simulink library "ee_lib_for_power_systems"  (R2025b) (continued)
== system ee_lib_for_power_systems/Power Converters/Thyristor Bridge (Three-Phase) ==
  g : Inport
  Demux : Demux  Outputs=6
  Simulink-PS Converter{,1..5} (6 alike) : nesl_utility/Simulink-PS Converter  PhysicalDomain=network_engine_domain  SubClassName=ps_input  LeftPortType=input  RightPortType=output  PseudoPeriodic=off  Frequencies=[]  NoiseDistribution=none  NoiseParameters=[]  Unit=1  AffineConversion=off  FilteringAndDerivatives=zero  SimscapeFilterOrder=1  InputFilterTimeConstant=0.001  UdotUserProvided=0
  Six-Pulse Gate Multiplexer : ee_lib/Semiconductors & Converters/Converters/Six-Pulse Gate Multiplexer  port_option=ee.enum.controlport.ps
  converter : ee_lib/Semiconductors & Converters/Converters/Converter (Three-Phase)  port_option=ee.enum.threePhasePort.composite  fidelity_option=ee.enum.converters.fidelity.detailed  device_type=ee.enum.converters.switchingdevice.thyristor  showThermalPort=false  K=0  Vth=0.5 V  Vgt=0.5 V  Vgt_off=0.4 V  Ih=1e-3 A  Vf=Vfs V  Ron=Ron Ohm  Rds=Ron Ohm  Goff=Goff 1/Ohm  Eon_scalar=22.86e-3 J  Eoff_scalar=17.14e-3 J  Eoff_natural_commutation=10e-3 J  Voff=300 V  Iout_scalar=600 A  onCurrentMeasDelay=0 s  useCurrentFromPreviousCycle=false  useVoltageFromPreviousCycle=false  diode_param=ee.enum.converters.protectiondiode.none  diode_Vf=Vfd V  diode_Ron=Ron Ohm  diode_Goff=Goff 1/Ohm  diode_C=50 nF  diode_iRM=-235 A  diode_iF=300 A  diode_idot=-50 A/us  diode_trr_param=ee.enum.converters.diodetrr.trr_factor  diode_trr_factor=3  diode_trr=15 us  diode_Qrr=1500 s*uA  diode_rec_loss_model=ee.enum.diode.reverseRecoveryModel.fixed  diode_ErecVal=0 J  diode_scaleWithIandV=false  diode_ErecMat=zeros(2, 3) J  diode_TjrecVec=[298.15, 398.15] K  diode_IfrecVec=[.1, 1, 10] A  diode_IrecVal=10 A  diode_VrecVal=10 V  diode_tFilter=1 / 1000 / 100 s  thermal_network_parameterization=ee.enum.thermalNetworkTopology.external  Rth=[.08, .5] K/W  Rth_Cauer=[.08, .1, .5] K/W  Rth_Foster=[.08, .14, .22, .16] K/W  thermal_mass_parameterization=ee.enum.thermalNetwork.timeconstant  thermal_mass=[.01, .5] J/K  thermal_mass_Cauer=[.01, .1, .5] J/K  thermal_mass_Foster=[.001, .005, .05, .5] J/K  thermal_time_constant=[.001, .2] s  thermal_time_constant_Cauer=[.001, .1, .2] s  thermal_time_constant_Foster=[7e-05, .0007, .01, .08] s  thermal_mass_initial_temperatures=[25, 25] degC  thermal_mass_initial_temperatures_Cauer=[25, 25, 25] degC  junction_thermal_mass=0.01 J/K  Tvec_initial_Foster=[25, 25, 25, 25] degC  snubber_type=ee.enum.converters.snubber.exclude  Cs=1e-7 F  Rs=0.1 Ohm  Vce_loss=[0, 150, 300] V
  + : PMIOPort  Side=Right
  "~ " : PMIOPort  Port=2  Side=Left
  - : PMIOPort  Port=3  Side=Right
  wires:
  Demux/6 -> Simulink-PS Converter2/1
  Demux/5 -> Simulink-PS Converter5/1
  Demux/4 -> Simulink-PS Converter/1
  Demux/3 -> Simulink-PS Converter3/1
  Demux/2 -> Simulink-PS Converter4/1
  Demux/1 -> Simulink-PS Converter1/1
  g/1 -> Demux/1
  connections:
  Simulink-PS Converter2/RConn1 -- Six-Pulse Gate Multiplexer/LConn3
  Simulink-PS Converter5/RConn1 -- Six-Pulse Gate Multiplexer/LConn6
  Simulink-PS Converter1/RConn1 -- Six-Pulse Gate Multiplexer/LConn2
  converter/LConn2 -- "~ "/RConn1
  Simulink-PS Converter3/RConn1 -- Six-Pulse Gate Multiplexer/LConn4
  Simulink-PS Converter/RConn1 -- Six-Pulse Gate Multiplexer/LConn1
  Simulink-PS Converter4/RConn1 -- Six-Pulse Gate Multiplexer/LConn5
  converter/LConn1 -- Six-Pulse Gate Multiplexer/RConn1
  -/RConn1 -- converter/RConn1
  +/RConn1 -- converter/RConn2

== system ee_lib_for_power_systems/Power Converters/Two-Level Average-Switch ==
  g : Inport
  Average-Value Voltage Source Converter (Three-Phase) : ee_lib/Semiconductors & Converters/Converters/Average-Value Voltage Source Converter (Three-Phase)  port_option=ee.enum.threePhasePort.composite  frequency_option=ee.enum.converters.frequency.variable  input_option=ee.enum.converters.inputOption.ModWave  FRated=60 Hz  losses_parameterization=ee.enum.converters.losses.fixed  Pfixed=1 W  ks=0.01  kc1=1.7 V  kc2=0.01 Ohm  Ploss_vect=[100, 800, 1870, 3250, 4900] W  Irms_vect=[10, 100, 200, 300, 400] A  Vdc_nom=300 V  thermal_mass=30000 J/K  temperature_specify=off  temperature_priority=High  temperature=298.15 K  temperature_nominal_specify=off  temperature_nominal_value=1  temperature_nominal_unit=K
      settings: InternalSimscapePortConfiguration="{\"Bottom\":[],\"Left\":[{\"id\":\"ModWave\",\"label\":\"ModWave\",\"type\":\"input\"},{\"id\":\"N\",\"label\":\"~\",\"type\":\"foundation.electrical.three_phase\"}],\"Right\":[{\"id\":\"p\",\"label\":\"+\",\"type\":\"foundation.electrical.electrical\"},{\"id\":\"n\",\"label\":\"-\",\"type\":\"foundation.electrical.electrical\"}],\"Top\":[]}"
  Demux : Demux  Outputs=6
  Mux : Mux  Inputs=3
  Simulink-PS Converter : nesl_utility/Simulink-PS Converter  PhysicalDomain=network_engine_domain  SubClassName=ps_input  LeftPortType=input  RightPortType=output  PseudoPeriodic=off  Frequencies=[]  NoiseDistribution=none  NoiseParameters=[]  Unit=1  AffineConversion=off  FilteringAndDerivatives=provide  SimscapeFilterOrder=1  InputFilterTimeConstant=0.001  UdotUserProvided=0
  Sum : Sum  Inputs=|+-
  Sum1 : Sum  Inputs=|+-
  Sum2 : Sum  Inputs=|+-
  + : PMIOPort  Side=Left
  - : PMIOPort  Port=2  Side=Left
  ~ : PMIOPort  Port=3  Side=Right
  wires:
  Mux/1 -> Simulink-PS Converter/1
  Sum2/1 -> Mux/3
  Sum1/1 -> Mux/2
  Sum/1 -> Mux/1
  Demux/6 -> Sum2/2
  Demux/5 -> Sum2/1
  Demux/4 -> Sum1/2
  Demux/3 -> Sum1/1
  Demux/2 -> Sum/2
  Demux/1 -> Sum/1
  g/1 -> Demux/1
  connections:
  Simulink-PS Converter/RConn1 -- Average-Value Voltage Source Converter (Three-Phase)/LConn1
  ~/RConn1 -- Average-Value Voltage Source Converter (Three-Phase)/LConn2
  +/RConn1 -- Average-Value Voltage Source Converter (Three-Phase)/RConn1
  -/RConn1 -- Average-Value Voltage Source Converter (Three-Phase)/RConn2

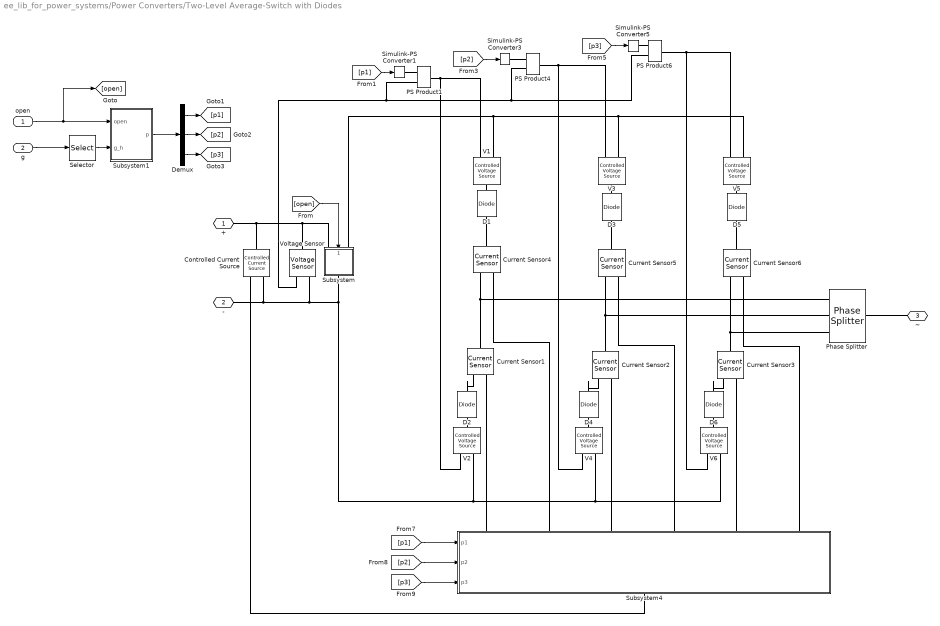
== system ee_lib_for_power_systems/Power Converters/Two-Level Average-Switch with Diodes ==
  open : Inport
  g : Inport  Port=2
  Controlled Current Source : fl_lib/Electrical/Electrical Sources/Controlled Current Source
  Current Sensor{1..6} (6 alike) : fl_lib/Electrical/Electrical Sensors/Current Sensor
  D{1..6} (6 alike) : fl_lib/Electrical/Electrical Elements/Diode  Vf=Vf V  Ron=Ron Ohm  Goff=Goff 1/Ohm  i_specify=off  i_priority=None  i=0 A  i_nominal_specify=off  i_nominal_value=1  i_nominal_unit=A  v_specify=off  v_priority=None  v=0 V  v_nominal_specify=off  v_nominal_value=1  v_nominal_unit=V
  Demux : Demux  Outputs=3
  PS Product1 : fl_lib/Physical Signals/Functions/PS Product
  PS Product4 : fl_lib/Physical Signals/Functions/PS Product
  PS Product6 : fl_lib/Physical Signals/Functions/PS Product
  Phase Splitter : ee_lib/Connectors & References/Phase Splitter
  Selector : Selector  InputPortWidth=6  IndexOptions=Index vector (dialog)  Indices=[1 3 5]  OutputSizes=1
  Simulink-PS Converter1 : nesl_utility/Simulink-PS Converter  PhysicalDomain=network_engine_domain  SubClassName=ps_input  LeftPortType=input  RightPortType=output  PseudoPeriodic=off  Frequencies=[]  NoiseDistribution=none  NoiseParameters=[]  Unit=1  AffineConversion=off  FilteringAndDerivatives=provide  SimscapeFilterOrder=1  InputFilterTimeConstant=0.001  UdotUserProvided=0
  Simulink-PS Converter3 : nesl_utility/Simulink-PS Converter  PhysicalDomain=network_engine_domain  SubClassName=ps_input  LeftPortType=input  RightPortType=output  PseudoPeriodic=off  Frequencies=[]  NoiseDistribution=none  NoiseParameters=[]  Unit=1  AffineConversion=off  FilteringAndDerivatives=provide  SimscapeFilterOrder=1  InputFilterTimeConstant=0.001  UdotUserProvided=0
  Simulink-PS Converter5 : nesl_utility/Simulink-PS Converter  PhysicalDomain=network_engine_domain  SubClassName=ps_input  LeftPortType=input  RightPortType=output  PseudoPeriodic=off  Frequencies=[]  NoiseDistribution=none  NoiseParameters=[]  Unit=1  AffineConversion=off  FilteringAndDerivatives=provide  SimscapeFilterOrder=1  InputFilterTimeConstant=0.001  UdotUserProvided=0
  Subsystem : Subsystem
      settings: PortSchema="{\"entries\":[{\"content\":{\"sides\":[{\"content\":{},\"type\":\"ConnectorPlacement.EquallySpacedRectSide\",\"uuid\":\"88db1bd8-f64b-4165-8d2c-7cfa4014cba2\"},{\"content\":{\"side\":\"RIGHT\"},\"type\":\"ConnectorPlacement.EquallySpacedRectSide\",\"uuid\":\"19f0c499-9eed-48d3-a8f5-fe9dad581b85\"},{\"content\":{\"connectorIds\":[\"LConn1\",\"In1\",\"LConn2\"],\"side\":\"TOP\"},\"type\":\"ConnectorPlacement.EquallySpacedRectSide\",\"uuid\":\"71d5fe89-ffc2-4430-819d-512bfd197a1c\"},{\"content\":{\"connectorIds\":[\"RConn1\"],\"side\":\"BOTTOM\"},\"type\":\"ConnectorPlacement.EquallySpacedRectSide\",\"uuid\":\"b828cb58-5f78-4b23-b8a0-147c7a9d81e7\"}]},\"type\":\"ConnectorPlacement.EquallySpacedConnector\",\"uuid\":\"64b174d4-80a0-4eb7-9a75-e3677787263f\"}],\"packageUris\":[\"http://schema.mathworks.com/mf0/Connector/R2020b\"],\"version\":\"1.0\"}"
  Subsystem1 : Subsystem
  Subsystem4 : Subsystem
      settings: PortSchema="{\"entries\":[{\"content\":{\"sides\":[{\"content\":{\"connectorIds\":[\"LConn1\",\"LConn2\",\"LConn3\",\"LConn4\",\"LConn5\",\"LConn6\"]},\"type\":\"ConnectorPlacement.EquallySpacedRectSide\",\"uuid\":\"5fd9aafb-b495-44e2-9499-8cdfe2ab4f56\"},{\"content\":{\"connectorIds\":[\"RConn1\"],\"side\":\"RIGHT\"},\"type\":\"ConnectorPlacement.EquallySpacedRectSide\",\"uuid\":\"0d8ceae3-09aa-4016-97de-ee2249771a91\"},{\"content\":{\"connectorIds\":[\"In1\",\"In2\",\"In3\"],\"side\":\"TOP\"},\"type\":\"ConnectorPlacement.EquallySpacedRectSide\",\"uuid\":\"0b4c8ea8-b524-451d-8365-47bbe880e547\"}]},\"type\":\"ConnectorPlacement.EquallySpacedConnector\",\"uuid\":\"44c8dc65-a8bd-4e02-863f-9a87b0ac0f96\"}],\"packageUris\":[\"http://schema.mathworks.com/mf0/Connector/R2020b\"],\"version\":\"1.0\"}"
  V{1..6} (6 alike) : fl_lib/Electrical/Electrical Sources/Controlled Voltage Source
  Voltage Sensor : fl_lib/Electrical/Electrical Sensors/Voltage Sensor
  + : PMIOPort  Side=Left
  - : PMIOPort  Port=2  Side=Left
  ~ : PMIOPort  Port=3  Side=Right
  wires:
  open/1 -> [open], Subsystem1/1(open)
  g/1 -> Selector/1
  Selector/1 -> Subsystem1/2(g_h)
  Subsystem1/1(p) -> Demux/1
  [open] -> Subsystem/1(open)
  Demux/1 -> [p1]
  Demux/2 -> [p2]
  Demux/3 -> [p3]
  [p1] -> Simulink-PS Converter1/1
  [p2] -> Simulink-PS Converter3/1
  [p3] -> Simulink-PS Converter5/1
  [p1] -> Subsystem4/1(p1)
  [p2] -> Subsystem4/2(p2)
  [p3] -> Subsystem4/3(p3)
  connections:
  Subsystem/LConn1 -- Controlled Current Source/LConn1 -- +/RConn1 -- Voltage Sensor/LConn1
  D2/LConn1 -- V2/LConn1
  PS Product1/RConn1 -- V2/RConn1 -- V1/RConn1
  Simulink-PS Converter1/RConn1 -- PS Product1/LConn1
  D2/RConn1 -- Current Sensor1/RConn2
  PS Product1/LConn2 -- Voltage Sensor/RConn1 -- PS Product6/LConn2 -- PS Product4/LConn2
  Current Sensor1/LConn1 -- Current Sensor4/RConn2 -- Phase Splitter/RConn1
  V1/LConn1 -- D1/RConn1
  Subsystem/RConn1 -- V6/RConn2 -- V4/RConn2 -- V2/RConn2 -- Controlled Current Source/RConn2 -- -/RConn1 -- Voltage Sensor/RConn2
  Subsystem/LConn2 -- V5/RConn2 -- V3/RConn2 -- V1/RConn2
  Phase Splitter/RConn2 -- Current Sensor5/RConn2 -- Current Sensor2/LConn1
  D3/RConn1 -- V3/LConn1
  D4/RConn1 -- Current Sensor2/RConn2
  Simulink-PS Converter3/RConn1 -- PS Product4/LConn1
  D4/LConn1 -- V4/LConn1
  PS Product4/RConn1 -- V3/RConn1 -- V4/RConn1
  Phase Splitter/RConn3 -- Current Sensor6/RConn2 -- Current Sensor3/LConn1
  D6/LConn1 -- V6/LConn1
  D6/RConn1 -- Current Sensor3/RConn2
  PS Product6/RConn1 -- V6/RConn1 -- V5/RConn1
  Simulink-PS Converter5/RConn1 -- PS Product6/LConn1
  D5/RConn1 -- V5/LConn1
  ~/RConn1 -- Phase Splitter/LConn1
  Current Sensor4/LConn1 -- D1/LConn1
  D3/LConn1 -- Current Sensor5/LConn1
  D5/LConn1 -- Current Sensor6/LConn1
  Subsystem4/LConn2 -- Current Sensor4/RConn1
  Subsystem4/LConn4 -- Current Sensor5/RConn1
  Subsystem4/LConn6 -- Current Sensor6/RConn1
  Subsystem4/RConn1 -- Controlled Current Source/RConn1
  Subsystem4/LConn1 -- Current Sensor1/RConn1
  Subsystem4/LConn3 -- Current Sensor2/RConn1
  Subsystem4/LConn5 -- Current Sensor3/RConn1

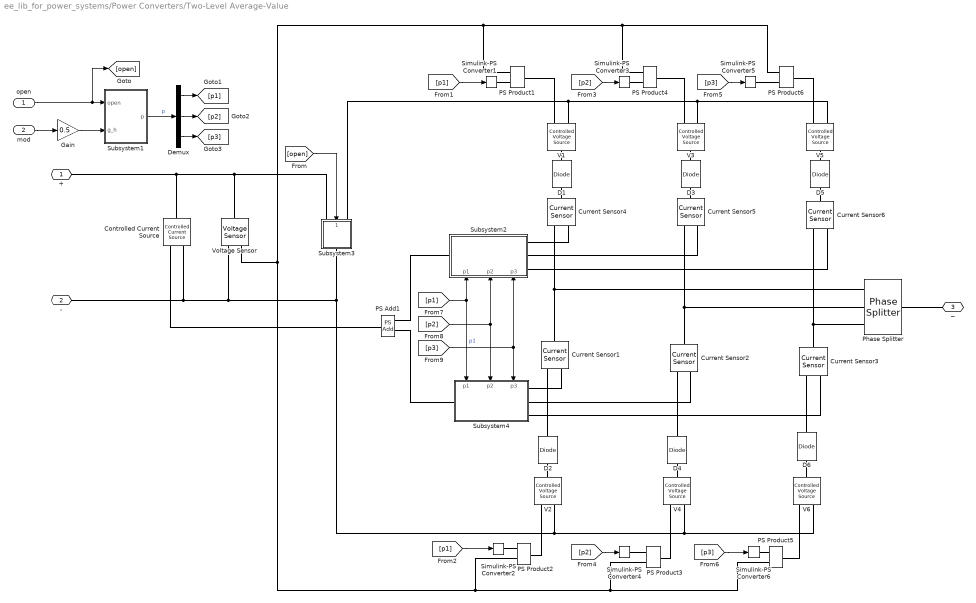
== system ee_lib_for_power_systems/Power Converters/Two-Level Average-Value ==
  open : Inport
  mod : Inport  Port=2
  Controlled Current Source : fl_lib/Electrical/Electrical Sources/Controlled Current Source
  Current Sensor{1..6} (6 alike) : fl_lib/Electrical/Electrical Sensors/Current Sensor
  D{1..6} (6 alike) : fl_lib/Electrical/Electrical Elements/Diode  Vf=Vf V  Ron=Ron Ohm  Goff=Goff 1/Ohm  i_specify=off  i_priority=None  i=0 A  i_nominal_specify=off  i_nominal_value=1  i_nominal_unit=A  v_specify=off  v_priority=None  v=0 V  v_nominal_specify=off  v_nominal_value=1  v_nominal_unit=V
  Demux : Demux  Outputs=3
  Gain : Gain  Gain=0.5
  PS Add1 : fl_lib/Physical Signals/Functions/PS Add
  PS Product{1..6} (6 alike) : fl_lib/Physical Signals/Functions/PS Product
  Phase Splitter : ee_lib/Connectors & References/Phase Splitter
  Simulink-PS Converter{1..6} (6 alike) : nesl_utility/Simulink-PS Converter  PhysicalDomain=network_engine_domain  SubClassName=ps_input  LeftPortType=input  RightPortType=output  PseudoPeriodic=off  Frequencies=[]  NoiseDistribution=none  NoiseParameters=[]  Unit=1  AffineConversion=off  FilteringAndDerivatives=provide  SimscapeFilterOrder=1  InputFilterTimeConstant=0.001  UdotUserProvided=0
  Subsystem1 : Subsystem
  Subsystem2 : Subsystem
      settings: PortSchema="{\"entries\":[{\"content\":{\"sides\":[{\"content\":{\"connectorIds\":[\"LConn1\",\"LConn2\",\"LConn3\"]},\"type\":\"ConnectorPlacement.EquallySpacedRectSide\",\"uuid\":\"5fd9aafb-b495-44e2-9499-8cdfe2ab4f56\"},{\"content\":{\"connectorIds\":[\"RConn1\"],\"side\":\"RIGHT\"},\"type\":\"ConnectorPlacement.EquallySpacedRectSide\",\"uuid\":\"0d8ceae3-09aa-4016-97de-ee2249771a91\"},{\"content\":{\"side\":\"TOP\"},\"type\":\"ConnectorPlacement.EquallySpacedRectSide\",\"uuid\":\"0b4c8ea8-b524-451d-8365-47bbe880e547\"},{\"content\":{\"connectorIds\":[\"In1\",\"In2\",\"In3\"],\"side\":\"BOTTOM\"},\"type\":\"ConnectorPlacement.EquallySpacedRectSide\",\"uuid\":\"91c10802-5411-4afb-b6a0-ea8a924d9d4e\"}]},\"type\":\"ConnectorPlacement.EquallySpacedConnector\",\"uuid\":\"44c8dc65-a8bd-4e02-863f-9a87b0ac0f96\"}],\"packageUris\":[\"http://schema.mathworks.com/mf0/Connector/R2020b\"],\"version\":\"1.0\"}"
  Subsystem3 : Subsystem
      settings: PortSchema="{\"entries\":[{\"content\":{\"sides\":[{\"content\":{},\"type\":\"ConnectorPlacement.EquallySpacedRectSide\",\"uuid\":\"88db1bd8-f64b-4165-8d2c-7cfa4014cba2\"},{\"content\":{\"side\":\"RIGHT\"},\"type\":\"ConnectorPlacement.EquallySpacedRectSide\",\"uuid\":\"19f0c499-9eed-48d3-a8f5-fe9dad581b85\"},{\"content\":{\"connectorIds\":[\"LConn1\",\"In1\",\"LConn2\"],\"side\":\"TOP\"},\"type\":\"ConnectorPlacement.EquallySpacedRectSide\",\"uuid\":\"71d5fe89-ffc2-4430-819d-512bfd197a1c\"},{\"content\":{\"connectorIds\":[\"RConn1\"],\"side\":\"BOTTOM\"},\"type\":\"ConnectorPlacement.EquallySpacedRectSide\",\"uuid\":\"b828cb58-5f78-4b23-b8a0-147c7a9d81e7\"}]},\"type\":\"ConnectorPlacement.EquallySpacedConnector\",\"uuid\":\"64b174d4-80a0-4eb7-9a75-e3677787263f\"}],\"packageUris\":[\"http://schema.mathworks.com/mf0/Connector/R2020b\"],\"version\":\"1.0\"}"
  Subsystem4 : Subsystem
      settings: PortSchema="{\"entries\":[{\"content\":{\"sides\":[{\"content\":{\"connectorIds\":[\"LConn1\",\"LConn2\",\"LConn3\"]},\"type\":\"ConnectorPlacement.EquallySpacedRectSide\",\"uuid\":\"5fd9aafb-b495-44e2-9499-8cdfe2ab4f56\"},{\"content\":{\"connectorIds\":[\"RConn1\"],\"side\":\"RIGHT\"},\"type\":\"ConnectorPlacement.EquallySpacedRectSide\",\"uuid\":\"0d8ceae3-09aa-4016-97de-ee2249771a91\"},{\"content\":{\"connectorIds\":[\"In1\",\"In2\",\"In3\"],\"side\":\"TOP\"},\"type\":\"ConnectorPlacement.EquallySpacedRectSide\",\"uuid\":\"0b4c8ea8-b524-451d-8365-47bbe880e547\"}]},\"type\":\"ConnectorPlacement.EquallySpacedConnector\",\"uuid\":\"44c8dc65-a8bd-4e02-863f-9a87b0ac0f96\"}],\"packageUris\":[\"http://schema.mathworks.com/mf0/Connector/R2020b\"],\"version\":\"1.0\"}"
  V{1..6} (6 alike) : fl_lib/Electrical/Electrical Sources/Controlled Voltage Source
  Voltage Sensor : fl_lib/Electrical/Electrical Sensors/Voltage Sensor
  + : PMIOPort  Side=Left
  - : PMIOPort  Port=2  Side=Left
  ~ : PMIOPort  Port=3  Side=Right
  wires:
  [p3] -> Simulink-PS Converter6/1
  [p2] -> Simulink-PS Converter4/1
  [p1] -> Simulink-PS Converter2/1
  [p3] -> Simulink-PS Converter5/1
  [p2] -> Simulink-PS Converter3/1
  [p1] -> Simulink-PS Converter1/1
  Demux/3 -> [p3]
  Demux/2 -> [p2]
  Demux/1 -> [p1]
  [open] -> Subsystem3/1(open)
  Subsystem1/1(p) -> Demux/1  "p"
  mod/1 -> Gain/1
  open/1 -> Subsystem1/1(open), [open]
  [p1] -> Subsystem2/3(p1), Subsystem4/3(p1)  "p1"
  [p2] -> Subsystem2/2(p2), Subsystem4/2(p2)
  [p3] -> Subsystem2/1(p3), Subsystem4/1(p3)
  Gain/1 -> Subsystem1/2(g_h)
  connections:
  Subsystem2/RConn1 -- PS Add1/LConn2
  Subsystem4/RConn1 -- PS Add1/LConn1
  Voltage Sensor/RConn2 -- -/RConn1 -- Controlled Current Source/RConn2 -- Subsystem3/RConn1 -- V4/RConn2 -- V6/RConn2 -- V2/RConn2
  PS Product6/LConn2 -- Simulink-PS Converter5/RConn1
  Simulink-PS Converter3/RConn1 -- PS Product4/LConn2
  Simulink-PS Converter1/RConn1 -- PS Product1/LConn2
  PS Product5/RConn1 -- V6/RConn1
  PS Product3/RConn1 -- V4/RConn1
  PS Product2/RConn1 -- V2/RConn1
  Simulink-PS Converter6/RConn1 -- PS Product5/LConn1
  Simulink-PS Converter2/RConn1 -- PS Product2/LConn1
  Simulink-PS Converter4/RConn1 -- PS Product3/LConn1
  Subsystem4/LConn3 -- Current Sensor3/RConn1
  Subsystem4/LConn2 -- Current Sensor2/RConn1
  Subsystem4/LConn1 -- Current Sensor1/RConn1
  D5/LConn1 -- Current Sensor6/LConn1
  D3/LConn1 -- Current Sensor5/LConn1
  Current Sensor4/LConn1 -- D1/LConn1
  D5/RConn1 -- V5/LConn1
  PS Product6/RConn1 -- V5/RConn1
  D6/RConn1 -- Current Sensor3/RConn2
  D6/LConn1 -- V6/LConn1
  Current Sensor3/LConn1 -- Current Sensor6/RConn2 -- Phase Splitter/RConn3
  PS Product4/RConn1 -- V3/RConn1
  D4/LConn1 -- V4/LConn1
  D4/RConn1 -- Current Sensor2/RConn2
  D3/RConn1 -- V3/LConn1
  Current Sensor2/LConn1 -- Current Sensor5/RConn2 -- Phase Splitter/RConn2
  V1/LConn1 -- D1/RConn1
  Current Sensor4/RConn2 -- Current Sensor1/LConn1 -- Phase Splitter/RConn1
  D2/RConn1 -- Current Sensor1/RConn2
  PS Product1/RConn1 -- V1/RConn1
  D2/LConn1 -- V2/LConn1
  Voltage Sensor/LConn1 -- +/RConn1 -- Controlled Current Source/LConn1 -- Subsystem3/LConn1
  Subsystem2/LConn1 -- Current Sensor4/RConn1
  Current Sensor5/RConn1 -- Subsystem2/LConn2
  Subsystem2/LConn3 -- Current Sensor6/RConn1
  PS Add1/RConn1 -- Controlled Current Source/RConn1
  V3/RConn2 -- V5/RConn2 -- Subsystem3/LConn2 -- V1/RConn2
  ~/RConn1 -- Phase Splitter/LConn1
  PS Product5/LConn2 -- PS Product3/LConn2 -- PS Product1/LConn1 -- PS Product4/LConn1 -- PS Product6/LConn1 -- Voltage Sensor/RConn1 -- PS Product2/LConn2

== system ee_lib_for_power_systems/Sense and Measure/Power sensor (Three-Phase) ==
  PS-Simulink Converter : nesl_utility/PS-Simulink Converter  PhysicalDomain=network_engine_domain  SubClassName=ps_output  LeftPortType=input  RightPortType=output  PseudoPeriodic=off  Frequencies=[]  VectorFormat=inherit  Unit=inherit  AffineConversion=off
  PS-Simulink Converter1 : nesl_utility/PS-Simulink Converter  PhysicalDomain=network_engine_domain  SubClassName=ps_output  LeftPortType=input  RightPortType=output  PseudoPeriodic=off  Frequencies=[]  VectorFormat=inherit  Unit=inherit  AffineConversion=off
  Power Sensor (Three-Phase) : ee_lib/Sensors & Transducers/Power Sensor (Three-Phase)  load_type=ee.enum.passive.type_balanced.balanced  f0=60 Hz
      settings: InternalSimscapePortConfiguration="{\"Bottom\":[],\"Left\":[{\"id\":\"N1\",\"label\":\"~1\",\"type\":\"foundation.electrical.three_phase\"}],\"Right\":[{\"id\":\"N2\",\"label\":\"~2\",\"type\":\"foundation.electrical.three_phase\"}],\"Top\":[{\"id\":\"Pm\",\"label\":\"P\",\"type\":\"output\"},{\"id\":\"Qm\",\"label\":\"Q\",\"type\":\"output\"}]}"
  ~1 : PMIOPort  Side=Left
  ~2 : PMIOPort  Port=2  Side=Right
  P : Outport
  Q : Outport  Port=2
  wires:
  PS-Simulink Converter1/1 -> Q/1
  PS-Simulink Converter/1 -> P/1
  connections:
  PS-Simulink Converter/LConn1 -- Power Sensor (Three-Phase)/LConn2
  Power Sensor (Three-Phase)/LConn1 -- ~1/RConn1
  PS-Simulink Converter1/LConn1 -- Power Sensor (Three-Phase)/LConn3
  Power Sensor (Three-Phase)/RConn1 -- ~2/RConn1

== system ee_lib_for_power_systems/Sense and Measure/VI sensor (Three-Phase) ==
  PS-Simulink Converter : nesl_utility/PS-Simulink Converter  PhysicalDomain=network_engine_domain  SubClassName=ps_output  LeftPortType=input  RightPortType=output  PseudoPeriodic=off  Frequencies=[]  VectorFormat=inherit  Unit=inherit  AffineConversion=off
  PS-Simulink Converter1 : nesl_utility/PS-Simulink Converter  PhysicalDomain=network_engine_domain  SubClassName=ps_output  LeftPortType=input  RightPortType=output  PseudoPeriodic=off  Frequencies=[]  VectorFormat=inherit  Unit=inherit  AffineConversion=off
  current_voltage : ee_lib/Sensors & Transducers/Current and Voltage Sensor (Three-Phase)  vMeasurementType=ee.enum.vmeasurement.lg  outputUnit=ee.enum.unit.si  SRated=Pnom A*V  VRated=Vnom V  I_specify=off  I_priority=None  I=[0, 0, 0] A  I_nominal_specify=off  I_nominal_value=1  I_nominal_unit=A  V_specify=off  V_priority=None  V=[0, 0, 0] V  V_nominal_specify=off  V_nominal_value=1  V_nominal_unit=V
      settings: InternalSimscapePortConfiguration="{\"Bottom\":[],\"Left\":[{\"id\":\"N1\",\"label\":\"~1\",\"type\":\"foundation.electrical.three_phase\"}],\"Right\":[{\"id\":\"V_output\",\"label\":\"V\",\"type\":\"output\"},{\"id\":\"I_output\",\"label\":\"I\",\"type\":\"output\"},{\"id\":\"N2\",\"label\":\"~2\",\"type\":\"foundation.electrical.three_phase\"}],\"Top\":[]}"
  ~1 : PMIOPort  Side=Left
  ~2 : PMIOPort  Port=2  Side=Right
  Vabc : Outport
  Iabc : Outport  Port=2
  wires:
  PS-Simulink Converter1/1 -> Iabc/1
  PS-Simulink Converter/1 -> Vabc/1
  connections:
  current_voltage/RConn3 -- ~2/RConn1
  PS-Simulink Converter/LConn1 -- current_voltage/RConn1
  current_voltage/LConn1 -- ~1/RConn1
  PS-Simulink Converter1/LConn1 -- current_voltage/RConn2

== system ee_lib_for_power_systems/Sense and Measure/Vectorized PQ meas ==
  Iabc : Inport
  Vabc : Inport  Port=2
  Product1 : Product  InputSameDT=on  SaturateOnIntegerOverflow=on  OutDataTypeStr=Inherit: Same as first input
  Product3 : Product  InputSameDT=on  SaturateOnIntegerOverflow=on  OutDataTypeStr=Inherit: Same as first input
  Sum1 : Sum  Inputs=1  CollapseMode=Specified dimension  InputSameDT=on  SaturateOnIntegerOverflow=on  OutDataTypeStr=Inherit: Same as first input
  Sum2 : Sum  Inputs=1  CollapseMode=Specified dimension  InputSameDT=on  SaturateOnIntegerOverflow=on  OutDataTypeStr=Inherit: Same as first input
  Sum4 : Sum  Inputs=+-  InputSameDT=on  SaturateOnIntegerOverflow=on  OutDataTypeStr=Inherit: Same as first input
  pu->V : Gain  Gain=1/sqrt(3)  SaturateOnIntegerOverflow=on  OutDataTypeStr=Inherit: Same as input  ParamDataTypeStr=Inherit: Same as input
  pu->V1 : Gain  Gain=[0 1 0;0 0 1;1 0 0]  Multiplication=Matrix(K*u)  SaturateOnIntegerOverflow=on  OutDataTypeStr=Inherit: Same as input  ParamDataTypeStr=Inherit: Same as input
  pu->V2 : Gain  Gain=[0 1 0;0 0 1;1 0 0]  Multiplication=Matrix(K*u)  SaturateOnIntegerOverflow=on  OutDataTypeStr=Inherit: Same as input  ParamDataTypeStr=Inherit: Same as input
  P : Outport  VectorParamsAs1DForOutWhenUnconnected=off
  Q : Outport  Port=2  VectorParamsAs1DForOutWhenUnconnected=off
  wires:
  pu->V/1 -> Q/1
  Sum1/1 -> pu->V/1
  Product3/1 -> Sum1/1
  Product1/1 -> Sum2/1
  Vabc/1 -> Sum4/1, pu->V1/1, Product1/1  "VV1"
  Iabc/1 -> Product3/2, Product1/2
  Sum2/1 -> P/1
  pu->V1/1 -> Sum4/2
  Sum4/1 -> pu->V2/1  "VV2"
  pu->V2/1 -> Product3/1

== system ee_lib_for_power_systems/Sense and Measure/current sensor ==
  Current Sensor : fl_lib/Electrical/Electrical Sensors/Current Sensor
  PS-Simulink Converter : nesl_utility/PS-Simulink Converter  PhysicalDomain=network_engine_domain  SubClassName=ps_output  LeftPortType=input  RightPortType=output  PseudoPeriodic=off  Frequencies=[]  VectorFormat=inherit  Unit=inherit  AffineConversion=off
  + : PMIOPort  Side=Left
  - : PMIOPort  Port=2  Side=Right
  i : Outport
  wires:
  PS-Simulink Converter/1 -> i/1
  connections:
  PS-Simulink Converter/LConn1 -- Current Sensor/RConn1
  Current Sensor/RConn2 -- -/RConn1
  Current Sensor/LConn1 -- +/RConn1
